simulink model "l1_ver116"  (Simulink 7.9) (continued)
== system l1_ver116 == (continued)
      mask init: same as l1_ver116/adcsnap0
      mask StyleString: popup(bram|dram),popup(1|2|3|4),popup(200|250|266|300|333),edit,popup(8|16|32|64|128),checkbox,checkbox,checkbox,checkbox
      mask CallbackString: snapshot_callback();||||||||
  gain : Subsystem  Tag=xps:sw_reg  AncestorBlock=xps_library/software register  mask{numios=1, io_dir=From Processor, io_delay=0, sample_period=1, name1=reg, bitwidth1=32, arith_type1=Unsigned, bin_pt1=0}
      mask prompts: numios "Num I/Os"; io_dir "I/O direction"; io_delay "I/O delay"; sample_period "Sample period"; name1 "Name"; bitwidth1 "Bitwidth"; arith_type1 "Type"; bin_pt1 "Binary pt"
      mask init:
      | swreg_init(gcb);
      mask StyleString: edit,popup(To Processor|From Processor),edit,edit,edit,edit,popup(Unsigned|Signed  (2's comp)|Boolean),edit
      mask CallbackString:
      | t = timer('StartDelay', 0.1); t.TimerFcn = {@swreg_cb, gcb}; start(t);|a = get_param(gcb, 'MaskEnables');
      | if strcmp(get_param(gcb, 'io_dir'), 'To Processor'),
      | a(4) = {'off'};
      | else
      | a(4) = {'on'};
      | end
      | set_param(gcb, 'MaskEnables', a);
      | |||||a = get_param(gcb, 'MaskEnables');
      | if strcmp(get_param(gcb, 'arith_type1'), 'Boolean'),
      | set_param(gcb, 'bitwidth1', '1');
      | set_param(gcb, 'bin_pt1', '0');
      | a(6) = {'off'};
      | a(8) = {'off'};
      | else
      | a(6) = {'on'};
      | a(8) = {'on'};
      | end
      | set_param(gcb, 'MaskEnables', a);
      | |
      mask TabNameString: Setup,Setup,Setup,Setup,IO1,IO1,IO1,IO1
  gbe0 : xps_library/ten_Gbe_v2  Tag=xps:tengbe_v2  flavour=sfp+  slot=1  port_r1=0  port_r2_cx4=0  port_r2_sfpp=0  rx_dist_ram=off  large_frames=on  show_param=on  pre_emph_r2=0.74  pre_emph=3  post_emph_r2=0.18  rxeqmix_r2=7  swing_r2=940  swing=800  fab_en=on  fab_mac=hex2dec('123456780000')  fab_ip=192*(2^24) + 168*(2^16) + 5*(2^8) + 20*(2^0)  fab_udp=10000  fab_gate=1  cpu_rx_en=on  cpu_tx_en=on
  gbe0_overflow : Subsystem  Tag=xps:sw_reg  AncestorBlock=xps_library/software register  mask{numios=1, io_dir=To Processor, io_delay=0, sample_period=1, name1=reg, bitwidth1=32, arith_type1=Unsigned, bin_pt1=0}
      mask prompts: numios "Num I/Os"; io_dir "I/O direction"; io_delay "I/O delay"; sample_period "Sample period"; name1 "Name"; bitwidth1 "Bitwidth"; arith_type1 "Type"; bin_pt1 "Binary pt"
      mask init:
      | swreg_init(gcb);
      mask StyleString: edit,popup(To Processor|From Processor),edit,edit,edit,edit,popup(Unsigned|Signed  (2's comp)|Boolean),edit
      mask CallbackString:
      | t = timer('StartDelay', 0.1); t.TimerFcn = {@swreg_cb, gcb}; start(t);|a = get_param(gcb, 'MaskEnables');
      | if strcmp(get_param(gcb, 'io_dir'), 'To Processor'),
      | a(4) = {'off'};
      | else
      | a(4) = {'on'};
      | end
      | set_param(gcb, 'MaskEnables', a);
      | |||||a = get_param(gcb, 'MaskEnables');
      | if strcmp(get_param(gcb, 'arith_type1'), 'Boolean'),
      | set_param(gcb, 'bitwidth1', '1');
      | set_param(gcb, 'bin_pt1', '0');
      | a(6) = {'off'};
      | a(8) = {'off'};
      | else
      | a(6) = {'on'};
      | a(8) = {'on'};
      | end
      | set_param(gcb, 'MaskEnables', a);
      | |
      mask TabNameString: Setup,Setup,Setup,Setup,IO1,IO1,IO1,IO1
  gbtlib : Subsystem  AncestorBlock=gbt_library/gen_rcs  mask(gen_rcs){lib_name=gbt_library, lib_src=git}
      mask prompts: lib_name "Library name"; lib_src "source of revision info"
      mask help:
      | Embeds revision control information for the user design and libraries.
      | On failure to locate specified revision information, falls back to
      | timestamps (current time for libraries).
      | A user specified value is also stored.
      mask init:
      | gen_rcs_init(gcb, ...
      |     'lib_name', lib_name, ...
      |     'lib_src', lib_src);
      settings: AttributesFormatString=|
      | /export/home/ptcs/scratch/models/gjones/vegas_devel/lib/gbt_library
      | FF7FA90 : dirty
      mask StyleString: edit,popup(timestamp|git|svn)
  latch : Subsystem
  latch1 : Subsystem
  latch10 : Subsystem
  latch11 : Subsystem
  latch12 : Subsystem
  latch13 : Subsystem
  latch14 : Subsystem
  latch15 : Subsystem
  latch2 : Subsystem
  latch3 : Subsystem
  latch4 : Subsystem
  latch5 : Subsystem
  latch6 : Subsystem
  latch7 : Subsystem
  latch8 : Subsystem
  latch9 : Subsystem
  mixer_addr1 : xbsIndex_r4/Counter  cnt_type=Free Running  cnt_to=Inf  operation=Up  start_count=0  cnt_by_val=1  arith_type=Unsigned  n_bits=11  bin_pt=0  load_pin=off  rst=on  en=off  explicit_period=on  period=1  dbl_ovrd=off  use_behavioral_HDL=off  implementation=Fabric  block_type=counter
      settings: block_version=11.4
  mixer_cnt : Subsystem  Tag=xps:sw_reg  AncestorBlock=xps_library/software register  mask{numios=1, io_dir=From Processor, io_delay=0, sample_period=1, name1=reg, bitwidth1=32, arith_type1=Unsigned, bin_pt1=0}
      mask prompts: numios "Num I/Os"; io_dir "I/O direction"; io_delay "I/O delay"; sample_period "Sample period"; name1 "Name"; bitwidth1 "Bitwidth"; arith_type1 "Type"; bin_pt1 "Binary pt"
      mask init:
      | swreg_init(gcb);
      mask StyleString: edit,popup(To Processor|From Processor),edit,edit,edit,edit,popup(Unsigned|Signed  (2's comp)|Boolean),edit
      mask CallbackString:
      | t = timer('StartDelay', 0.1); t.TimerFcn = {@swreg_cb, gcb}; start(t);|a = get_param(gcb, 'MaskEnables');
      | if strcmp(get_param(gcb, 'io_dir'), 'To Processor'),
      | a(4) = {'off'};
      | else
      | a(4) = {'on'};
      | end
      | set_param(gcb, 'MaskEnables', a);
      | |||||a = get_param(gcb, 'MaskEnables');
      | if strcmp(get_param(gcb, 'arith_type1'), 'Boolean'),
      | set_param(gcb, 'bitwidth1', '1');
      | set_param(gcb, 'bin_pt1', '0');
      | a(6) = {'off'};
      | a(8) = {'off'};
      | else
      | a(6) = {'on'};
      | a(8) = {'on'};
      | end
      | set_param(gcb, 'MaskEnables', a);
      | |
      mask TabNameString: Setup,Setup,Setup,Setup,IO1,IO1,IO1,IO1
  negedge_delay : casper_library_misc/negedge_delay  pulse_len=2^20
  packetizer : Subsystem  mask{header_words=2, data_words=1024}
      mask prompts: header_words "Number of 64-bit words in header"; data_words "Number of 64-bit words in data"
  packsnap : Subsystem  Tag=casper:snapshot  AncestorBlock=casper_library_scopes/snapshot  mask(snapshot){storage=bram, dram_dimm=1, dram_clock=200, nsamples=12, data_width=64, offset=off, circap=off, value=off, use_dsp48=on}
      mask prompts: storage "Storage medium"; dram_dimm "DRAM dimm"; dram_clock "DRAM clock rate"; nsamples "Number of Samples (2^?)"; data_width "Data width"; offset "Start delay support"; circap "Circular capture support"; value "Extra value capture support"; use_dsp48 "Use DSP48s to implement counters"
      mask help:
      | Standard snap block, but with the following optional extras;
      |  1) Optionally delay the fabric trigger by a user-specified number of valids.
      |  2) Capture continuously after a trigger until a stop pulse is received. Data is captured into BRAM continuously, with old data being overwritten until a stop command is received. Then stop capturing when memory is filled. This enables you to get some data before and some data after the stop pulse. Use the 'tr_en_cnt' register to determine where you are in your vector. 'Addr' register's MSb now indicates 'busy capturing'.
      |  3) Optionally capture a value on an input port to a register at the same time as the first data sample.
      |  4) Implement counters using DSP48Es to save resources.
      mask init: same as l1_ver116/adcsnap0
      mask StyleString: popup(bram|dram),popup(1|2|3|4),popup(200|250|266|300|333),edit,popup(8|16|32|64|128),checkbox,checkbox,checkbox,checkbox
      mask CallbackString: snapshot_callback();||||||||
  rcs : Subsystem  AncestorBlock=casper_library_misc/rcs  mask(rcs){app_src=git, lib_src=git, user_val=2010116}
      mask prompts: app_src "Application revision info to capture"; lib_src "CASPER libraries revision info to capture"; user_val "User specified value"
      mask help:
      | Embeds revision control information for the user design and libraries.
      | On failure to locate specified revision information, falls back to
      | timestamps (current time for libraries).
      | A user specified value is also stored.
      mask init:
      | rcs_init(gcb, ...
      |     'app_src', app_src, ...
      |     'lib_src', lib_src);
      mask StyleString: popup(timestamp|git|svn),popup(timestamp|git|svn),edit
  reset : Subsystem  Tag=xps:sw_reg  AncestorBlock=xps_library/software register  mask{numios=1, io_dir=From Processor, io_delay=0, sample_period=1, name1=reg, bitwidth1=32, arith_type1=Unsigned, bin_pt1=0}
      mask prompts: numios "Num I/Os"; io_dir "I/O direction"; io_delay "I/O delay"; sample_period "Sample period"; name1 "Name"; bitwidth1 "Bitwidth"; arith_type1 "Type"; bin_pt1 "Binary pt"
      mask init:
      | swreg_init(gcb);
      mask StyleString: edit,popup(To Processor|From Processor),edit,edit,edit,edit,popup(Unsigned|Signed  (2's comp)|Boolean),edit
      mask CallbackString:
      | t = timer('StartDelay', 0.1); t.TimerFcn = {@swreg_cb, gcb}; start(t);|a = get_param(gcb, 'MaskEnables');
      | if strcmp(get_param(gcb, 'io_dir'), 'To Processor'),
      | a(4) = {'off'};
      | else
      | a(4) = {'on'};
      | end
      | set_param(gcb, 'MaskEnables', a);
      | |||||a = get_param(gcb, 'MaskEnables');
      | if strcmp(get_param(gcb, 'arith_type1'), 'Boolean'),
      | set_param(gcb, 'bitwidth1', '1');
      | set_param(gcb, 'bin_pt1', '0');
      | a(6) = {'off'};
      | a(8) = {'off'};
      | else
      | a(6) = {'on'};
      | a(8) = {'on'};
      | end
      | set_param(gcb, 'MaskEnables', a);
      | |
      mask TabNameString: Setup,Setup,Setup,Setup,IO1,IO1,IO1,IO1
  reshaper : Subsystem
  round1 : Subsystem
  round2 : Subsystem
  rshpsnap : Subsystem  Tag=casper:snapshot  AncestorBlock=casper_library_scopes/snapshot  mask(snapshot){storage=bram, dram_dimm=1, dram_clock=200, nsamples=12, data_width=64, offset=off, circap=off, value=off, use_dsp48=on}
      mask prompts: storage "Storage medium"; dram_dimm "DRAM dimm"; dram_clock "DRAM clock rate"; nsamples "Number of Samples (2^?)"; data_width "Data width"; offset "Start delay support"; circap "Circular capture support"; value "Extra value capture support"; use_dsp48 "Use DSP48s to implement counters"
      mask help:
      | Standard snap block, but with the following optional extras;
      |  1) Optionally delay the fabric trigger by a user-specified number of valids.
      |  2) Capture continuously after a trigger until a stop pulse is received. Data is captured into BRAM continuously, with old data being overwritten until a stop command is received. Then stop capturing when memory is filled. This enables you to get some data before and some data after the stop pulse. Use the 'tr_en_cnt' register to determine where you are in your vector. 'Addr' register's MSb now indicates 'busy capturing'.
      |  3) Optionally capture a value on an input port to a register at the same time as the first data sample.
      |  4) Implement counters using DSP48Es to save resources.
      mask init: same as l1_ver116/adcsnap0
      mask StyleString: popup(bram|dram),popup(1|2|3|4),popup(200|250|266|300|333),edit,popup(8|16|32|64|128),checkbox,checkbox,checkbox,checkbox
      mask CallbackString: snapshot_callback();||||||||
  sg : Subsystem
  ssg : Subsystem
  swapri : Subsystem
  swapri1 : Subsystem
  trig : Subsystem  Tag=xps:sw_reg  AncestorBlock=xps_library/software register  mask{numios=1, io_dir=From Processor, io_delay=0, sample_period=1, name1=reg, bitwidth1=32, arith_type1=Unsigned, bin_pt1=0}
      mask prompts: numios "Num I/Os"; io_dir "I/O direction"; io_delay "I/O delay"; sample_period "Sample period"; name1 "Name"; bitwidth1 "Bitwidth"; arith_type1 "Type"; bin_pt1 "Binary pt"
      mask init:
      | swreg_init(gcb);
      mask StyleString: edit,popup(To Processor|From Processor),edit,edit,edit,edit,popup(Unsigned|Signed  (2's comp)|Boolean),edit
      mask CallbackString:
      | t = timer('StartDelay', 0.1); t.TimerFcn = {@swreg_cb, gcb}; start(t);|a = get_param(gcb, 'MaskEnables');
      | if strcmp(get_param(gcb, 'io_dir'), 'To Processor'),
      | a(4) = {'off'};
      | else
      | a(4) = {'on'};
      | end
      | set_param(gcb, 'MaskEnables', a);
      | |||||a = get_param(gcb, 'MaskEnables');
      | if strcmp(get_param(gcb, 'arith_type1'), 'Boolean'),
      | set_param(gcb, 'bitwidth1', '1');
      | set_param(gcb, 'bin_pt1', '0');
      | a(6) = {'off'};
      | a(8) = {'off'};
      | else
      | a(6) = {'on'};
      | a(8) = {'on'};
      | end
      | set_param(gcb, 'MaskEnables', a);
      | |
      mask TabNameString: Setup,Setup,Setup,Setup,IO1,IO1,IO1,IO1
  xblocks : Subsystem  AncestorBlock=gbt_library/gen_rcs  mask(gen_rcs){lib_name=xblocks_devel, lib_src=git}
      mask prompts: lib_name "Library name"; lib_src "source of revision info"
      mask help:
      | Embeds revision control information for the user design and libraries.
      | On failure to locate specified revision information, falls back to
      | timestamps (current time for libraries).
      | A user specified value is also stored.
      mask init:
      | gen_rcs_init(gcb, ...
      |     'lib_name', lib_name, ...
      |     'lib_src', lib_src);
      settings: AttributesFormatString=|
      | /export/home/ptcs/scratch/models/gjones/xblocks_devel/xblocks_library
      | 8A521D5 : dirty
      mask StyleString: edit,popup(timestamp|git|svn)
  wires:
  mixer_addr1/1 -> Delay1/1, Relational/1
  Delay1/1 -> [mixer_addr]
  Relational/1 -> mixer_addr1/1
  mixer_cnt/1(in_reg) -> Relational/2
  Constant3/1 -> mixer_cnt/1(sim_reg)
  [cic_sync] -> Delay73/1
  Delay54/1 -> [pol1_a0]
  Delay67/1 -> [pol1_a2]
  Delay68/1 -> [pol1_a4]
  Delay69/1 -> [pol1_a6]
  Delay70/1 -> [pol1_a8]
  Delay74/1 -> [pol1_a10]
  Delay75/1 -> [pol1_a12]
  Delay76/1 -> [pol1_a14]
  Delay/1 -> converter/1(u8_0)
  Delay2/1 -> converter1/1(u8_0)
  Delay3/1 -> converter2/1(u8_0)
  Delay4/1 -> converter3/1(u8_0)
  Delay5/1 -> converter4/1(u8_0)
  Delay6/1 -> converter5/1(u8_0)
  Delay7/1 -> converter6/1(u8_0)
  Delay8/1 -> converter7/1(u8_0)
  converter/1(s8_7) -> Delay54/1
  converter1/1(s8_7) -> Delay67/1
  converter2/1(s8_7) -> Delay68/1
  converter3/1(s8_7) -> Delay69/1
  converter4/1(s8_7) -> Delay70/1
  converter5/1(s8_7) -> Delay74/1
  converter6/1(s8_7) -> Delay75/1
  converter7/1(s8_7) -> Delay76/1
  Counter/1 -> Slice2/1
  Slice2/1 -> edge_detect4/1
  edge_detect4/1 -> Delay84/1
  gbe0_overflow/1(sim_out) -> Terminator5/1
  Constant1/1 -> dest_ip/1(sim_reg)
  Constant7/1 -> dest_port/1(sim_reg)
  dest_ip/1(in_reg) -> [dest_ip]
  dest_port/1(in_reg) -> [dest_port]
  [dest_ip] -> gbe0/4
  [dest_port] -> Slice4/1
  packetizer/3(pkt_eof) -> gbe0/6, [pkt_eof]
  [overflow] -> gbe0_overflow/1(out_reg)
  [spead_rst] -> packetizer/1(reset)
  [status_bits] -> packetizer/2(status)
  reset/1(in_reg) -> [reset]  "reset"
  Slice4/1 -> gbe0/5
  gbe0/6 -> Terminator4/1
  gbe0/7 -> Terminator6/1
  gbe0/8 -> Terminator7/1
  gbe0/9 -> Terminator8/1
  gbe0/10 -> Terminator11/1
  gbe0/11 -> Terminator3/1
  Constant11/1 -> gbe0/7
  gbe0/1 -> Terminator14/1
  gbe0/2 -> Terminator15/1
  gbe0/3 -> Terminator16/1
  gbe0/4 -> Terminator17/1
  gbe0/5 -> negedge_delay/1
  Constant12/1 -> gbe0/8
  gbe0/12 -> Terminator12/1
  [gbe_rst] -> gbe0/1
  negedge_delay/1 -> [overflow]
  Constant13/1 -> Relational1/2
  Counter2/1 -> [valid_cntr], Relational1/1
  Relational1/1 -> [valid]  "valid"
  Constant10/1 -> reset/1(sim_reg)
  Constant31/1 -> Register18/1
  sg/2(pps_out) -> Register18/3, [master_rst1]
  Register18/1 -> Inverter2/1, Inverter1/1, [data_enable]  "data_enable"
  arm/1(in_reg) -> Slice8/1
  Pulse Generator/1 -> arm/1(sim_reg)
  Inverter1/1 -> sg/2(pps_en)
  edge_detect/1 -> Logical3/2
  Slice11/1 -> Logical3/1
  Slice9/1 -> Logical/2
  Logical3/1 -> Logical/3
  [arm] -> Register18/2
  [adc_sync] -> sg/1(pps)
  sg/1(sync_out) -> [sync_out]
  Inverter2/1 -> [spead_rst]  "spead_rst"
  Logical/1 -> [gbe_rst]  "gbe_rst"
  [overflow] -> edge_detect/1
  [reset] -> Slice9/1, Slice11/1
  Delay21/1 -> Delay85/1
  Slice10/1 -> edge_detect3/1
  edge_detect3/1 -> [adc_sync]  "adc_sync"
  [spead_rst] -> Counter2/1
  Slice8/1 -> [arm]  "arm"
  [arm] -> edge_detect6/1
  [valid] -> reshaper/1(valid)
  [data_enable] -> reshaper/2(data_enable)
  reshaper/1(data_out) -> packetizer/3(data), [snaprshp]
  reshaper/2(data_valid) -> packetizer/4(data_valid), [snaprshp_valid]
  Constant4/1 -> trig/1(sim_reg)
  trig/1(in_reg) -> Slice19/1
  packetizer/1(pkt_data) -> gbe0/2, [pktizer_dout]
  Concat2/1 -> Delay20/1
  Delay38/1 -> [pol2_a0]
  Delay40/1 -> [pol2_a2]
  Delay41/1 -> [pol2_a4]
  Delay42/1 -> [pol2_a6]
  Delay44/1 -> [pol2_a8]
  Delay45/1 -> [pol2_a10]
  Delay46/1 -> [pol2_a12]
  Delay47/1 -> [pol2_a14]
  Delay25/1 -> converter8/1(u8_0)
  Delay33/1 -> converter9/1(u8_0)
  Delay35/1 -> converter10/1(u8_0)
  Delay36/1 -> converter11/1(u8_0)
  Delay37/1 -> converter12/1(u8_0)
  Delay39/1 -> converter13/1(u8_0)
  Delay43/1 -> converter14/1(u8_0)
  Delay48/1 -> converter15/1(u8_0)
  converter8/1(s8_7) -> Delay38/1
  converter9/1(s8_7) -> Delay40/1
  converter10/1(s8_7) -> Delay41/1
  converter11/1(s8_7) -> Delay42/1
  converter12/1(s8_7) -> Delay44/1
  converter13/1(s8_7) -> Delay45/1
  converter14/1(s8_7) -> Delay46/1
  converter15/1(s8_7) -> Delay47/1
  asiaa_adc5g/2(a1) -> Delay9/1
  asiaa_adc5g/4(a3) -> Delay13/1
  asiaa_adc5g/6(a5) -> Delay15/1
  asiaa_adc5g/8(a7) -> Delay17/1
  asiaa_adc5g/10(a9) -> Delay26/1
  asiaa_adc5g/12(a11) -> Delay28/1
  asiaa_adc5g/14(a13) -> Delay29/1
  asiaa_adc5g/16(a15) -> Delay62/1
  asiaa_adc5g/1(a0) -> Delay/1
  asiaa_adc5g/15(a14) -> Delay8/1
  asiaa_adc5g/13(a12) -> Delay7/1
  asiaa_adc5g/11(a10) -> Delay6/1
  asiaa_adc5g/7(a6) -> Delay4/1
  asiaa_adc5g/5(a4) -> Delay3/1
  asiaa_adc5g/3(a2) -> Delay2/1
  asiaa_adc5g/9(a8) -> Delay5/1
  asiaa_adc5g/17(sync_out) -> Delay21/1
  asiaa_adc5g1/1(a0) -> Delay25/1
  asiaa_adc5g1/2(a1) -> Delay31/1
  asiaa_adc5g1/3(a2) -> Delay33/1
  asiaa_adc5g1/4(a3) -> Delay34/1
  asiaa_adc5g1/5(a4) -> Delay35/1
  asiaa_adc5g1/6(a5) -> Delay50/1
  asiaa_adc5g1/7(a6) -> Delay36/1
  asiaa_adc5g1/8(a7) -> Delay52/1
  asiaa_adc5g1/9(a8) -> Delay37/1
  asiaa_adc5g1/10(a9) -> Delay55/1
  asiaa_adc5g1/11(a10) -> Delay39/1
  asiaa_adc5g1/12(a11) -> Delay57/1
  asiaa_adc5g1/13(a12) -> Delay43/1
  asiaa_adc5g1/14(a13) -> Delay59/1
  asiaa_adc5g1/15(a14) -> Delay48/1
  asiaa_adc5g1/16(a15) -> Delay64/1
  Delay11/1 -> [pol1_a1]
  Delay9/1 -> converter16/1(u8_0)
  converter16/1(s8_7) -> Delay11/1
  Delay12/1 -> [pol1_a3]
  Delay13/1 -> converter17/1(u8_0)
  converter17/1(s8_7) -> Delay12/1
  Delay14/1 -> [pol1_a5]
  Delay15/1 -> converter18/1(u8_0)
  converter18/1(s8_7) -> Delay14/1
  Delay16/1 -> [pol1_a7]
  Delay17/1 -> converter19/1(u8_0)
  converter19/1(s8_7) -> Delay16/1
  Delay23/1 -> [pol1_a9]
  Delay26/1 -> converter20/1(u8_0)
  converter20/1(s8_7) -> Delay23/1
  Delay27/1 -> [pol1_a11]
  Delay28/1 -> converter21/1(u8_0)
  converter21/1(s8_7) -> Delay27/1
  Delay30/1 -> [pol1_a13]
  Delay29/1 -> converter22/1(u8_0)
  converter22/1(s8_7) -> Delay30/1
  Delay32/1 -> [pol2_a1]
  Delay31/1 -> converter23/1(u8_0)
  converter23/1(s8_7) -> Delay32/1
  Delay49/1 -> [pol2_a3]
  Delay34/1 -> converter24/1(u8_0)
  converter24/1(s8_7) -> Delay49/1
  Delay51/1 -> [pol2_a5]
  Delay50/1 -> converter25/1(u8_0)
  converter25/1(s8_7) -> Delay51/1
  Delay53/1 -> [pol2_a7]
  Delay52/1 -> converter26/1(u8_0)
  converter26/1(s8_7) -> Delay53/1
  Delay56/1 -> [pol2_a9]
  Delay55/1 -> converter27/1(u8_0)
  converter27/1(s8_7) -> Delay56/1
  Delay58/1 -> [pol2_a11]
  Delay57/1 -> converter28/1(u8_0)
  converter28/1(s8_7) -> Delay58/1
  Delay60/1 -> [pol2_a13]
  Delay59/1 -> converter29/1(u8_0)
  converter29/1(s8_7) -> Delay60/1
  Delay61/1 -> [pol1_a15]
  Delay62/1 -> converter30/1(u8_0)
  converter30/1(s8_7) -> Delay61/1
  Delay63/1 -> [pol2_a15]
  Delay64/1 -> converter31/1(u8_0)
  converter31/1(s8_7) -> Delay63/1
  Delay73/1 -> fastmixbyfour/1(sync_in)
  [pol1_a0] -> fastmixbyfour/2(In1)
  [pol1_a1] -> fastmixbyfour/3(In2)
  [pol1_a2] -> fastmixbyfour/4(In3)
  [pol1_a3] -> fastmixbyfour/5(In4)
  [pol1_a4] -> fastmixbyfour/6(In5)
  [pol1_a5] -> fastmixbyfour/7(In6)
  [pol1_a6] -> fastmixbyfour/8(In7)
  [pol1_a7] -> fastmixbyfour/9(In8)
  [pol1_a8] -> fastmixbyfour/10(In9)
  [pol1_a9] -> fastmixbyfour/11(In10)
  [pol1_a10] -> fastmixbyfour/12(In11)
  [pol1_a11] -> fastmixbyfour/13(In12)
  [pol1_a12] -> fastmixbyfour/14(In13)
  [pol1_a13] -> fastmixbyfour/15(In14)
  [pol1_a14] -> fastmixbyfour/16(In15)
  [pol1_a15] -> fastmixbyfour/17(In16)
  Constant23/1 -> adcsnap0/2(we)
  bus_create1/1(bus_out) -> adcsnap0/1(din)
  Constant24/1 -> adcsnap1/2(we)
  bus_create2/1(bus_out) -> adcsnap1/1(din)
  [pkt_valid] -> packsnap/2(we)
  [pktizer_dout] -> packsnap/1(din)
  Slice19/1 -> edge_detect2/1
  edge_detect2/1 -> [trig]
  [trig] -> adcsnap0/3(trig)
  [trig] -> adcsnap1/3(trig)
  [trig] -> packsnap/3(trig)
  fastmixbyfour1/1(sync_out) -> dec_fir1/1(sync_in)
  dec_fir3/2(dout) -> swapri/1(In1)
  [cic_sync] -> Delay83/1
  Delay83/1 -> fastmixbyfour1/1(sync_in)
  [pol2_a0] -> fastmixbyfour1/2(In1)
  [pol2_a1] -> fastmixbyfour1/3(In2)
  [pol2_a2] -> fastmixbyfour1/4(In3)
  [pol2_a3] -> fastmixbyfour1/5(In4)
  [pol2_a4] -> fastmixbyfour1/6(In5)
  [pol2_a5] -> fastmixbyfour1/7(In6)
  [pol2_a6] -> fastmixbyfour1/8(In7)
  [pol2_a7] -> fastmixbyfour1/9(In8)
  [pol2_a8] -> fastmixbyfour1/10(In9)
  [pol2_a9] -> fastmixbyfour1/11(In10)
  [pol2_a10] -> fastmixbyfour1/12(In11)
  [pol2_a11] -> fastmixbyfour1/13(In12)
  [pol2_a12] -> fastmixbyfour1/14(In13)
  [pol2_a13] -> fastmixbyfour1/15(In14)
  [pol2_a14] -> fastmixbyfour1/16(In15)
  [pol2_a15] -> fastmixbyfour1/17(In16)
  Constant5/1 -> filtersnap0/2(we)
  [snapfilter0] -> filtersnap0/1(din)
  [trig] -> filtersnap0/3(trig)
  Constant6/1 -> filtersnap1/2(we)
  [snapfilter1] -> filtersnap1/1(din)
  [trig] -> filtersnap1/3(trig)
  Delay84/1 -> [cic_sync]
  Delay85/1 -> Slice10/1
  [snaprshp_valid] -> rshpsnap/2(we)
  [snaprshp] -> rshpsnap/1(din)
  [trig] -> rshpsnap/3(trig)
  Constant/1 -> gain/1(sim_reg)
  gain/1(in_reg) -> Delay86/1
  Delay86/1 -> [gain]
  [gain] -> round2/2(Scale)
  round2/1(dOut) -> latch1/1(din), latch/1(din), latch14/1(din), latch2/1(din), latch3/1(din), latch4/1(din), latch5/1(din), [snapfilter0], latch6/1(din)
  [gain] -> round1/2(Scale)
  dec_fir1/2(dout) -> swapri1/1(In1)
  Constant8/1 -> filtersnap_nogain0/2(we)
  [trig] -> filtersnap_nogain0/3(trig)
  Constant14/1 -> filtersnap_nogain1/2(we)
  [trig] -> filtersnap_nogain1/3(trig)
  ssg/1(statusbits) -> [status_bits]
  [master_rst1] -> Register/1
  Register/1 -> ssg/1(reset)
  [spec_tick] -> ssg/2(spec_tick)
  [master_rst1] -> Counter1/1
  Counter1/1 -> Slice1/1
  Slice1/1 -> edge_detect5/1(in)
  edge_detect5/1(out) -> [spec_tick]
  [snapfilter1_nogain] -> filtersnap_nogain1/1(din)
  [snapfilter0_nogain] -> filtersnap_nogain0/1(din)
  Gateway Out/1 -> Scope/1
  Gateway Out1/1 -> Scope/2
  Pulse Generator1/1 -> asiaa_adc5g/2(sim_sync)
  Gateway Out2/1 -> Scope/3
  [pol1_6] -> Concat2/1
  [pol2_6] -> Concat2/2
  [pol1_7] -> Concat2/3
  [pol2_7] -> Concat2/4
  [pol1_4] -> Concat2/5
  [pol2_4] -> Concat2/6
  [pol1_5] -> Concat2/7
  [pol2_5] -> Concat2/8
  [pol1_2] -> Concat2/9
  [pol2_2] -> Concat2/10
  [pol1_3] -> Concat2/11
  [pol2_3] -> Concat2/12
  [pol1_0] -> Concat2/13
  [pol2_0] -> Concat2/14
  [pol1_1] -> Concat2/15
  [pol2_1] -> Concat2/16
  [valid_cntr] -> latch/2(cntr)
  [valid_cntr] -> latch1/2(cntr)
  [valid_cntr] -> latch2/2(cntr)
  [valid_cntr] -> latch3/2(cntr)
  [valid_cntr] -> latch4/2(cntr)
  [valid_cntr] -> latch5/2(cntr)
  [valid_cntr] -> latch6/2(cntr)
  [valid_cntr] -> latch7/2(cntr)
  [valid_cntr] -> latch8/2(cntr)
  [valid_cntr] -> latch9/2(cntr)
  [valid_cntr] -> latch10/2(cntr)
  [valid_cntr] -> latch11/2(cntr)
  [valid_cntr] -> latch12/2(cntr)
  [valid_cntr] -> latch13/2(cntr)
  latch6/1(dout) -> [pol1_0]
  latch5/1(dout) -> [pol1_1]
  latch4/1(dout) -> [pol1_2]
  latch3/1(dout) -> [pol1_3]
  latch2/1(dout) -> [pol1_4]
  latch1/1(dout) -> [pol1_5]
  latch/1(dout) -> [pol1_6]
  latch7/1(dout) -> [pol2_6]
  latch8/1(dout) -> [pol2_5]
  latch9/1(dout) -> [pol2_4]
  latch10/1(dout) -> [pol2_3]
  latch11/1(dout) -> [pol2_2]
  latch12/1(dout) -> [pol2_1]
  latch13/1(dout) -> [pol2_0]
  round1/1(dOut) -> latch8/1(din), latch7/1(din), latch15/1(din), latch9/1(din), latch10/1(din), latch11/1(din), latch12/1(din), latch13/1(din), [snapfilter1]
  [valid_cntr] -> latch14/2(cntr)
  latch14/1(dout) -> [pol1_7]
  [valid_cntr] -> latch15/2(cntr)
  latch15/1(dout) -> [pol2_7]
  Delay20/1 -> reshaper/3(data_in)
  [data_enable] -> Gateway Out1/1
  [snaprshp_valid] -> Gateway Out2/1
  [pol2_a0] -> bus_create2/1(in1)
  [pol2_a1] -> bus_create2/2(in2)
  [pol2_a2] -> bus_create2/3(in3)
  [pol2_a3] -> bus_create2/4(in4)
  [pol2_a4] -> bus_create2/5(in5)
  [pol2_a5] -> bus_create2/6(in6)
  [pol2_a6] -> bus_create2/7(in7)
  [pol2_a7] -> bus_create2/8(in8)
  [pol2_a8] -> bus_create2/9(in9)
  [pol2_a9] -> bus_create2/10(in10)
  [pol2_a10] -> bus_create2/11(in11)
  [pol2_a11] -> bus_create2/12(in12)
  [pol2_a12] -> bus_create2/13(in13)
  [pol2_a13] -> bus_create2/14(in14)
  [pol2_a14] -> bus_create2/15(in15)
  [pol2_a15] -> bus_create2/16(in16)
  [pol1_a0] -> bus_create1/1(in1)
  [pol1_a1] -> bus_create1/2(in2)
  [pol1_a2] -> bus_create1/3(in3)
  [pol1_a3] -> bus_create1/4(in4)
  [pol1_a4] -> bus_create1/5(in5)
  [pol1_a5] -> bus_create1/6(in6)
  [pol1_a6] -> bus_create1/7(in7)
  [pol1_a7] -> bus_create1/8(in8)
  [pol1_a8] -> bus_create1/9(in9)
  [pol1_a9] -> bus_create1/10(in10)
  [pol1_a10] -> bus_create1/11(in11)
  [pol1_a11] -> bus_create1/12(in12)
  [pol1_a12] -> bus_create1/13(in13)
  [pol1_a13] -> bus_create1/14(in14)
  [pol1_a14] -> bus_create1/15(in15)
  [pol1_a15] -> bus_create1/16(in16)
  [valid] -> Gateway Out/1
  packetizer/2(pkt_valid) -> Logical1/1
  [data_enable] -> Logical1/2
  Logical1/1 -> [pkt_valid], gbe0/3
  fastmixbyfour/1(sync_out) -> dec_fir3/1(sync_in)
  fastmixbyfour/2(r1) -> downshiftbyeight/1(In1)
  fastmixbyfour/3(i1) -> downshiftbyeight/2(In2)
  fastmixbyfour/4(r2) -> downshiftbyeight/3(In3)
  fastmixbyfour/5(i2) -> downshiftbyeight/4(In4)
  fastmixbyfour/6(r3) -> downshiftbyeight/5(In5)
  fastmixbyfour/7(i3) -> downshiftbyeight/6(In6)
  fastmixbyfour/8(r4) -> downshiftbyeight/7(In7)
  fastmixbyfour/9(i4) -> downshiftbyeight/8(In8)
  fastmixbyfour/10(r5) -> downshiftbyeight/9(In9)
  fastmixbyfour/11(i5) -> downshiftbyeight/10(In10)
  fastmixbyfour/12(r6) -> downshiftbyeight/11(In11)
  fastmixbyfour/13(i6) -> downshiftbyeight/12(In12)
  fastmixbyfour/14(r7) -> downshiftbyeight/13(In13)
  fastmixbyfour/15(i7) -> downshiftbyeight/14(In14)
  fastmixbyfour/16(r8) -> downshiftbyeight/15(In15)
  fastmixbyfour/17(i8) -> downshiftbyeight/16(In16)
  fastmixbyfour/18(r9) -> downshiftbyeight/17(In17)
  fastmixbyfour/19(i9) -> downshiftbyeight/18(In18)
  fastmixbyfour/20(r10) -> downshiftbyeight/19(In19)
  fastmixbyfour/21(i10) -> downshiftbyeight/20(In20)
  fastmixbyfour/22(r11) -> downshiftbyeight/21(In21)
  fastmixbyfour/23(i11) -> downshiftbyeight/22(In22)
  fastmixbyfour/24(r12) -> downshiftbyeight/23(In23)
  fastmixbyfour/25(i12) -> downshiftbyeight/24(In24)
  fastmixbyfour/26(r13) -> downshiftbyeight/25(In25)
  fastmixbyfour/27(i13) -> downshiftbyeight/26(In26)
  fastmixbyfour/28(r14) -> downshiftbyeight/27(In27)
  fastmixbyfour/29(i14) -> downshiftbyeight/28(In28)
  fastmixbyfour/30(r15) -> downshiftbyeight/29(In29)
  fastmixbyfour/31(i15) -> downshiftbyeight/30(In30)
  fastmixbyfour/32(r16) -> downshiftbyeight/31(In31)
  fastmixbyfour/33(i16) -> downshiftbyeight/32(In32)
  downshiftbyeight/1(Out1) -> dec_fir3/2(real1)
  downshiftbyeight/2(Out2) -> dec_fir3/3(imag1)
  downshiftbyeight/3(Out3) -> dec_fir3/4(real2)
  downshiftbyeight/4(Out4) -> dec_fir3/5(imag2)
  downshiftbyeight/5(Out5) -> dec_fir3/6(real3)
  downshiftbyeight/6(Out6) -> dec_fir3/7(imag3)
  downshiftbyeight/7(Out7) -> dec_fir3/8(real4)
  downshiftbyeight/8(Out8) -> dec_fir3/9(imag4)
  downshiftbyeight/9(Out9) -> dec_fir3/10(real5)
  downshiftbyeight/10(Out10) -> dec_fir3/11(imag5)
  downshiftbyeight/11(Out11) -> dec_fir3/12(real6)
  downshiftbyeight/12(Out12) -> dec_fir3/13(imag6)
  downshiftbyeight/13(Out13) -> dec_fir3/14(real7)
  downshiftbyeight/14(Out14) -> dec_fir3/15(imag7)
  downshiftbyeight/15(Out15) -> dec_fir3/16(real8)
  downshiftbyeight/16(Out16) -> dec_fir3/17(imag8)
  downshiftbyeight/17(Out17) -> dec_fir3/18(real9)
  downshiftbyeight/18(Out18) -> dec_fir3/19(imag9)
  downshiftbyeight/19(Out19) -> dec_fir3/20(real10)
  downshiftbyeight/20(Out20) -> dec_fir3/21(imag10)
  downshiftbyeight/21(Out21) -> dec_fir3/22(real11)
  downshiftbyeight/22(Out22) -> dec_fir3/23(imag11)
  downshiftbyeight/23(Out23) -> dec_fir3/24(real12)
  downshiftbyeight/24(Out24) -> dec_fir3/25(imag12)
  downshiftbyeight/25(Out25) -> dec_fir3/26(real13)
  downshiftbyeight/26(Out26) -> dec_fir3/27(imag13)
  downshiftbyeight/27(Out27) -> dec_fir3/28(real14)
  downshiftbyeight/28(Out28) -> dec_fir3/29(imag14)
  downshiftbyeight/29(Out29) -> dec_fir3/30(real15)
  downshiftbyeight/30(Out30) -> dec_fir3/31(imag15)
  downshiftbyeight/31(Out31) -> dec_fir3/32(real16)
  downshiftbyeight/32(Out32) -> dec_fir3/33(imag16)
  fastmixbyfour1/2(r1) -> downshiftbyeight1/1(In1)
  fastmixbyfour1/3(i1) -> downshiftbyeight1/2(In2)
  fastmixbyfour1/4(r2) -> downshiftbyeight1/3(In3)
  fastmixbyfour1/5(i2) -> downshiftbyeight1/4(In4)
  fastmixbyfour1/6(r3) -> downshiftbyeight1/5(In5)
  fastmixbyfour1/7(i3) -> downshiftbyeight1/6(In6)
  fastmixbyfour1/8(r4) -> downshiftbyeight1/7(In7)
  fastmixbyfour1/9(i4) -> downshiftbyeight1/8(In8)
  fastmixbyfour1/10(r5) -> downshiftbyeight1/9(In9)
  fastmixbyfour1/11(i5) -> downshiftbyeight1/10(In10)
  fastmixbyfour1/12(r6) -> downshiftbyeight1/11(In11)
  fastmixbyfour1/13(i6) -> downshiftbyeight1/12(In12)
  fastmixbyfour1/14(r7) -> downshiftbyeight1/13(In13)
  fastmixbyfour1/15(i7) -> downshiftbyeight1/14(In14)
  fastmixbyfour1/16(r8) -> downshiftbyeight1/15(In15)
  fastmixbyfour1/17(i8) -> downshiftbyeight1/16(In16)
  fastmixbyfour1/18(r9) -> downshiftbyeight1/17(In17)
  fastmixbyfour1/19(i9) -> downshiftbyeight1/18(In18)
  fastmixbyfour1/20(r10) -> downshiftbyeight1/19(In19)
  fastmixbyfour1/21(i10) -> downshiftbyeight1/20(In20)
  fastmixbyfour1/22(r11) -> downshiftbyeight1/21(In21)
  fastmixbyfour1/23(i11) -> downshiftbyeight1/22(In22)
  fastmixbyfour1/24(r12) -> downshiftbyeight1/23(In23)
  fastmixbyfour1/25(i12) -> downshiftbyeight1/24(In24)
  fastmixbyfour1/26(r13) -> downshiftbyeight1/25(In25)
  fastmixbyfour1/27(i13) -> downshiftbyeight1/26(In26)
  fastmixbyfour1/28(r14) -> downshiftbyeight1/27(In27)
  fastmixbyfour1/29(i14) -> downshiftbyeight1/28(In28)
  fastmixbyfour1/30(r15) -> downshiftbyeight1/29(In29)
  fastmixbyfour1/31(i15) -> downshiftbyeight1/30(In30)
  fastmixbyfour1/32(r16) -> downshiftbyeight1/31(In31)
  fastmixbyfour1/33(i16) -> downshiftbyeight1/32(In32)
  downshiftbyeight1/1(Out1) -> dec_fir1/2(real1)
  downshiftbyeight1/2(Out2) -> dec_fir1/3(imag1)
  downshiftbyeight1/3(Out3) -> dec_fir1/4(real2)
  downshiftbyeight1/4(Out4) -> dec_fir1/5(imag2)
  downshiftbyeight1/5(Out5) -> dec_fir1/6(real3)
  downshiftbyeight1/6(Out6) -> dec_fir1/7(imag3)
  downshiftbyeight1/7(Out7) -> dec_fir1/8(real4)
  downshiftbyeight1/8(Out8) -> dec_fir1/9(imag4)
  downshiftbyeight1/9(Out9) -> dec_fir1/10(real5)
  downshiftbyeight1/10(Out10) -> dec_fir1/11(imag5)
  downshiftbyeight1/11(Out11) -> dec_fir1/12(real6)
  downshiftbyeight1/12(Out12) -> dec_fir1/13(imag6)
  downshiftbyeight1/13(Out13) -> dec_fir1/14(real7)
  downshiftbyeight1/14(Out14) -> dec_fir1/15(imag7)
  downshiftbyeight1/15(Out15) -> dec_fir1/16(real8)
  downshiftbyeight1/16(Out16) -> dec_fir1/17(imag8)
  downshiftbyeight1/17(Out17) -> dec_fir1/18(real9)
  downshiftbyeight1/18(Out18) -> dec_fir1/19(imag9)
  downshiftbyeight1/19(Out19) -> dec_fir1/20(real10)
  downshiftbyeight1/20(Out20) -> dec_fir1/21(imag10)
  downshiftbyeight1/21(Out21) -> dec_fir1/22(real11)
  downshiftbyeight1/22(Out22) -> dec_fir1/23(imag11)
  downshiftbyeight1/23(Out23) -> dec_fir1/24(real12)
  downshiftbyeight1/24(Out24) -> dec_fir1/25(imag12)
  downshiftbyeight1/25(Out25) -> dec_fir1/26(real13)
  downshiftbyeight1/26(Out26) -> dec_fir1/27(imag13)
  downshiftbyeight1/27(Out27) -> dec_fir1/28(real14)
  downshiftbyeight1/28(Out28) -> dec_fir1/29(imag14)
  downshiftbyeight1/29(Out29) -> dec_fir1/30(real15)
  downshiftbyeight1/30(Out30) -> dec_fir1/31(imag15)
  downshiftbyeight1/31(Out31) -> dec_fir1/32(real16)
  downshiftbyeight1/32(Out32) -> dec_fir1/33(imag16)
  edge_detect6/1 -> Delay10/1
  Delay10/1 -> Logical/1
  swapri/1(Out1) -> [snapfilter0_nogain], round2/1(dIn)
  swapri1/1(Out1) -> round1/1(dIn), [snapfilter1_nogain]
